simulink model "ofdm_txrx_mimo"  (Simulink 7.1)
settings: Solver=ode45  StartTime=0.0  StopTime=6e3  RelTol=1e-3
PreLoadFcn:
  | ofdm_rx_mimo_init
InitFcn:
  | ofdm_rx_mimo_init
StartFcn:
  | ofdm_rx_mimo_init
values from neighbouring files (symbols this model uses):
  BER_RAM_init_size = 2048    [ofdm_tx_mimo_init.m]
  CPLength = 16    [ofdm_tx_mimo_init.m]
  CRCPolynomial = hex2dec('04c11db7')    [ofdm_tx_mimo_init.m]
  freqOffset_track_filtCoef_i = hex2dec('2500')/2^32    [ofdm_rx_mimo_init.m]
  freqOffset_track_filtCoef_p = hex2dec('d0000')/2^32    [ofdm_rx_mimo_init.m]
  hi = zeros(1,length(cci))    [ofdm_rx_mimo_init.m]
  hr = zeros(1,length(ccr))    [ofdm_rx_mimo_init.m]
  max_CP_length = 16    [ofdm_tx_mimo_init.m]
  max_num_baseRateSymbols = 31    [ofdm_tx_mimo_init.m]
  max_num_subcarriers = 64    [ofdm_tx_mimo_init.m]
  max_num_trainingSymbols = 15    [ofdm_tx_mimo_init.m]
  max_numBytes = 16384    [ofdm_tx_mimo_init.m]
  max_OFDM_symbols = 2047    [ofdm_tx_mimo_init.m]
  modConstellation_qam16 = .75*[1 3 -1 -3]./3    [ofdm_tx_mimo_init.m]
  modConstellation_qam256 = 0.9375*[3 1 5 7 11 9 13 15 -3 -1 -5 -7 -11 -9 -13 -15]./15    [ofdm_tx_mimo_init.m]
  modConstellation_qam64 = 0.875*[3 1 5 7 -3 -1 -5 -7]./7    [ofdm_tx_mimo_init.m]
  modConstellation_qpsk = [1 -1]./sqrt(2)    [ofdm_tx_mimo_init.m]
  numBaseRateSymbols = 2    [ofdm_tx_mimo_init.m]
  numSubcarriers = 64    [ofdm_tx_mimo_init.m]
  numTrainingSymbols = 2    [ofdm_tx_mimo_init.m]
  pilot1_indicies = 7 + ( (64-7) * 2^16)    (= 3735559)    [ofdm_tx_mimo_init.m]
  pilot2_indicies = 21 + ( (64-21) * 2^16)    (= 2818069)    [ofdm_tx_mimo_init.m]
  pilotValue_neg = hex2dec('8000') + (2^16 * 0)    [ofdm_tx_mimo_init.m]
  pilotValue_pos = hex2dec('7FFF') + (2^16 * 0)    [ofdm_tx_mimo_init.m]
  pkt_crossCorr_thresh = 0.7    [ofdm_rx_mimo_init.m]
  pkt_energy_thresh = 0    [ofdm_rx_mimo_init.m]
  pkt_numPayloadBytes = 1400+42-6    (= 1436)    [ofdm_tx_mimo_init.m]
  pktByteNums = numHeaderBytes +  				byteIndex_numPayloadBytes(1)*2^8 +  				byteIndex_numPayloadBytes(2)*2^16 +  				byteIndex_simpleDynModMasks * 2^24    [ofdm_rx_mimo_init.m]
  PktDet_Delay = 26 + Symbol_Timing_Offset*2^7    (= 1050)    [ofdm_rx_mimo_init.m]
  preamble = 6*[0 shortsyms_10 longsyms_2]    [ofdm_tx_mimo_init.m]
  preamble_I = real(preamble)    [ofdm_tx_mimo_init.m]
  preamble_Q = imag(preamble)    [ofdm_tx_mimo_init.m]
  RAM_init_size = max_numBytes    (= 16384)    [ofdm_tx_mimo_init.m]
  reg_Interrupt_PktBuf_Control = reg_InterruptControl +  	1 * 2^16 +  	0 * 2^24    (= 65656)    [ofdm_rx_mimo_init.m]
  rx_controlBits = 1 * 2  		 + 1 * 4  		 + 0 * 8  		 + 1 * 16 * rx_SISO_Mode  		 + 1 * 32  		 + 1 * 64  		 + 0 * 128  		 + 1 * 256  		 + 0 * 512  		 + 1 * 1024  		 + 0 * 2048  		 + 1 * 4096  		 + 1 * 8192  		 + 0 * 16384  		 + 1 * 65536  		 + 1 * 131072  		 + 0 * 262144  		 + 1 * 524288    [ofdm_rx_mimo_init.m]
  Rx_PhaseNoiseTrack_K = 0.6094    [ofdm_rx_mimo_init.m]
  rx_postEq_scaling = round(rxScaling*2^11) * (1 + 2^16)    [ofdm_rx_mimo_init.m]
  RxDataScrambling_Seq = [40 198 78 63 82 173 102 245 48 111 172 115 147 230 216 93 72 65 62 2 205 242 122 90 128 83 105 97 73 10 5 252]    [ofdm_tx_mimo_init.m]
  simOnly_numSamples = length(preamble)+( (numSubcarriers+CPLength)*(numTrainingSymbols + numBaseRateSymbols + numFullRateSymbols/2) )    [ofdm_tx_mimo_init.m]
  train = [train train]    [ofdm_tx_mimo_init.m]
  tx_controlBits = (antB_preambleShift * 2^4) + (2^0 * tx_SISO_Mode) + 4*0 + 8*1    (= 73)    [ofdm_tx_mimo_init.m]
  TxDataScrambling_Seq = [40 198 78 63 82 173 102 245 48 111 172 115 147 230 216 93 72 65 62 2 205 242 122 90 128 83 105 97 73 10 5 252]    [ofdm_tx_mimo_init.m]
  txReg_symbolCounts = (2^16 * numFullRateSymbols) + (2^8 *numBaseRateSymbols ) + numTrainingSymbols    [ofdm_tx_mimo_init.m]
  TxRx_FFTScaling = bin2dec('010111') + (bin2dec('000101') * 2^6)    [ofdm_tx_mimo_init.m]
undefined symbols (not defined in the file or in its folder): AntA, AntB, BaseRate, CRC_table_gen, gcbh, intfilt, pktTiming_count_payloadStart, pktTiming_symbol_length, xlBlockLoadCallback, xlGetSimulinkPeriod
libraries: xbsIndex_r4 x4212, dspmlti4 x4, dspsigops x4, commchan3 x2, simulink x2, commrflib2 x2, dspsrcs4 x1
(part 3 of 43)

== system ofdm_txrx_mimo/Memory-mapped Registers ==
  Rx Registers : Subsystem
  Static Parameters (might be registers someday) : Subsystem
  Tx Registers : Subsystem
  TxRx Registers : Subsystem

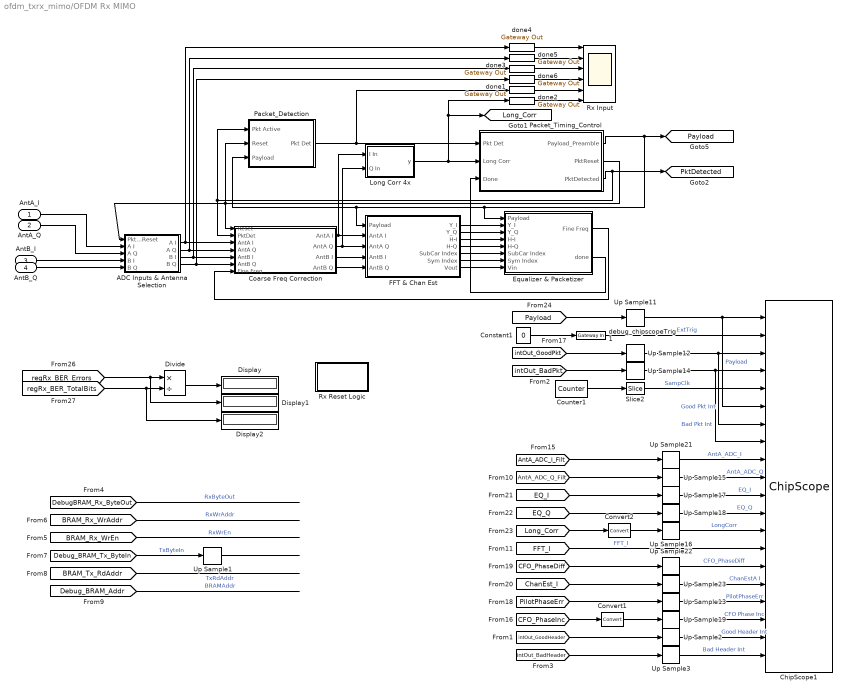
== system ofdm_txrx_mimo/OFDM Rx MIMO ==
  AntA_I : Inport  OutDataType=sfix(16)  OutScaling=2^0
  AntA_Q : Inport  Port=2  OutDataType=sfix(16)  OutScaling=2^0
  AntB_I : Inport  Port=3  OutDataType=sfix(16)  OutScaling=2^0
  AntB_Q : Inport  Port=4  OutDataType=sfix(16)  OutScaling=2^0
  ADC Inputs & Antenna Selection : Subsystem
  ChipScope1 : xbsIndex_r4/ChipScope  num_trig_ports=5  current_port=4  show_trig_port=0  match_units=1  match_type=Basic with edges  data_is_trigger=off  num_data_ports=15  data_depth=4096  SRL16s=on  match_type_t0=2  match_type_t1=2  match_type_t2=2  match_type_t3=2  match_type_t4=2  match_type_t5=1  match_type_t6=1  match_type_t7=1  match_type_t8=1  match_type_t9=1  match_type_t10=1  match_type_t11=1  match_type_t12=1  match_type_t13=1  match_type_t14=1  match_type_t15=1  match_units_t0=1  match_units_t1=1  match_units_t2=1  match_units_t3=1  match_units_t4=1  match_units_t5=1  match_units_t6=1  match_units_t7=1  match_units_t8=1  match_units_t9=1  match_units_t10=1  match_units_t11=1  match_units_t12=1  match_units_t13=1  match_units_t14=1  match_units_t15=1  dbl_ovrd=0  block_type=chipscope
      settings: infoedit="Enables run-time debugging and verification of signals by inserting ChipScope Pro ICON and ILA cores.<br><br>  Restrictions: <br>Only one ChipScope core can be instantiated in a System Generator design.This block cannot be used at the same time as JTAG hardware co-simulation.A design or subsystem containing a ChipScope block must have at least one output port."  block_version=VER_STRING_GOES_HERE
  Coarse Freq Correction : Subsystem
  Constant1 : Constant  Value=0  OutDataType=sfix(16)  OutScaling=2^0
  Convert1 : xbsIndex_r4/Convert  arith_type=Unsigned  n_bits=20  bin_pt=20  quantization=Truncate  overflow=Wrap  latency=0  dbl_ovrd=off  pipeline=off  block_type=convert
      settings: xl_area=[0 0 0 0 0 0 0]  block_version=VER_STRING_GOES_HERE
  Convert2 : xbsIndex_r4/Convert  arith_type=Unsigned  n_bits=14  bin_pt=0  quantization=Truncate  overflow=Wrap  latency=0  dbl_ovrd=off  pipeline=off  block_type=convert
      settings: xl_area=[0 0 0 0 0 0 0]  block_version=VER_STRING_GOES_HERE
  Counter1 : xbsIndex_r4/Counter  cnt_type=Free Running  cnt_to=Inf  operation=Up  start_count=0  cnt_by_val=1  arith_type=Unsigned  n_bits=2  bin_pt=0  load_pin=off  rst=off  en=off  explicit_period=on  period=1  dbl_ovrd=off  use_behavioral_HDL=off  use_rpm=on  implementation=Fabric  block_type=counter
      settings: xl_area=[5 10 0 10 0 0 0]  block_version=VER_STRING_GOES_HERE
  Display : Display
  Display1 : Display
  Display2 : Display
  Divide : Product  Inputs=*/  OutDataType=sfix(16)  OutScaling=2^-10
  Equalizer & Packetizer : Subsystem
  FFT & Chan Est : Subsystem
  Long Corr 4x : Subsystem
  Packet_Detection : Subsystem
  Packet_Timing_Control : Subsystem
  Rx Input : Scope  NumInputPorts=6
      settings: TimeRange=10000  YMin=-0.5~-0.4~-0.5~-0.4~0~0  YMax=0.5~0.4~0.5~0.4~1~7000  SaveName=ScopeData48
  Rx Reset Logic : Subsystem
  Slice2 : xbsIndex_r4/Slice  nbits=1  boolean_output=off  mode=Upper Bit Location + Width  bit1=0  base1=MSB of Input  bit0=0  base0=LSB of Input  dbl_ovrd=off  block_type=slice
      settings: infoedit="Extracts a given range of bits from each input sample and presents it at the output.  The output type is ordinarily unsigned with binary point at zero, but can be Boolean when the slice is one bit wide.<P><P>Hardware notes: In hardware this block costs nothing."  block_version=VER_STRING_GOES_HERE
  Up Sample{1..3,12..14,17..18,23} (9 alike) : xbsIndex_r4/Up Sample  sample_ratio=2  copy_samples=on  dbl_ovrd=off  block_type=usamp
      settings: infoedit="Up samples input data.  Inserted values  can be zeros or copies of the most recent input sample.<P><P>Hardware notes: No hardware is needed if inserted values are copies of the input sample; otherwise, a mux and single bit flip-flop are used."  block_version=VER_STRING_GOES_HERE
  Up Sample{11,15..16,19,21..22} (6 alike) : xbsIndex_r4/Up Sample  sample_ratio=4  copy_samples=on  dbl_ovrd=off  block_type=usamp
      settings: infoedit="Up samples input data.  Inserted values  can be zeros or copies of the most recent input sample.<P><P>Hardware notes: No hardware is needed if inserted values are copies of the input sample; otherwise, a mux and single bit flip-flop are used."  block_version=VER_STRING_GOES_HERE
  debug_chipscopeTrig1 : xbsIndex_r4/Gateway In  arith_type=Boolean  n_bits=14  bin_pt=13  quantization="Round  (unbiased: +/- Inf)"  overflow=Saturate  period=1  dbl_ovrd=off  timing_constraint=None  locs_specified=off  LOCs={}  block_type=gatewayin
      settings: infoedit="Gateway in block.  Converts inputs of type Simulink integer, double and fixed point to  Xilinx fixed point type.<P><P>Hardware notes:  In hardware these blocks become top level input ports."  block_version=VER_STRING_GOES_HERE
  done{1..6} (6 alike) : xbsIndex_r4/Gateway Out  hdl_port=off  timing_constraint=None  locs_specified=off  LOCs={}  block_type=gatewayout
      settings: infoedit="Gateway out block.  Converts Xilinx fixed point inputs into ouputs of type Simulink integer, double, or fixed point.<P><P>Hardware notes:  In hardware these blocks become top level output ports or are discarded, depending on how they are configured."  xl_area=[0 0 0 0 1 0 0]  block_version=VER_STRING_GOES_HERE
  tag scope: Long_Corr=global, Payload=global, PktDetected=global
  wires:
  Packet_Detection/1(Pkt Det) -> done1/1, Packet_Timing_Control/1(Pkt Det)
  Packet_Timing_Control/1(Payload_Preamble) -> Equalizer & Packetizer/1(Payload), FFT & Chan Est/1(Payload), Packet_Detection/3(Payload), [Payload]
  Long Corr 4x/1(y) -> Packet_Timing_Control/2(Long Corr), done2/1, [Long_Corr]
  done1/1 -> Rx Input/5
  done4/1 -> Rx Input/1
  done5/1 -> Rx Input/2
  done3/1 -> Rx Input/3
  done6/1 -> Rx Input/4
  Coarse Freq Correction/1("AntA I ") -> Long Corr 4x/1(I In), FFT & Chan Est/2(AntA I)
  Coarse Freq Correction/2("AntA Q ") -> Long Corr 4x/2(Q In), FFT & Chan Est/3(AntA Q)
  Coarse Freq Correction/3("AntB I ") -> FFT & Chan Est/4(AntB I)
  Coarse Freq Correction/4("AntB Q ") -> FFT & Chan Est/5(AntB Q)
  ADC Inputs & Antenna Selection/1(A I) -> Coarse Freq Correction/3(AntA I), done4/1
  ADC Inputs & Antenna Selection/2(A Q) -> Coarse Freq Correction/4(AntA Q), done5/1
  ADC Inputs & Antenna Selection/3(B I) -> Coarse Freq Correction/5(AntB I), done3/1
  ADC Inputs & Antenna Selection/4(B Q) -> Coarse Freq Correction/6(AntB Q), done6/1
  done2/1 -> Rx Input/6
  AntB_I/1 -> ADC Inputs & Antenna Selection/4("B I ")
  AntB_Q/1 -> ADC Inputs & Antenna Selection/5("B Q ")
  Equalizer & Packetizer/1(Fine Freq) -> Coarse Freq Correction/7(Fine Freq)
  Equalizer & Packetizer/2(done) -> Packet_Timing_Control/3(Done)
  FFT & Chan Est/1(Y_I) -> Equalizer & Packetizer/2(Y_I)
  FFT & Chan Est/2(Y_Q) -> Equalizer & Packetizer/3(Y_Q)
  FFT & Chan Est/3(H-I) -> Equalizer & Packetizer/4(H-I)
  FFT & Chan Est/4(H-Q) -> Equalizer & Packetizer/5(H-Q)
  FFT & Chan Est/5(SubCar Index) -> Equalizer & Packetizer/6(SubCar Index)
  FFT & Chan Est/6(Sym Index) -> Equalizer & Packetizer/7(Sym Index)
  FFT & Chan Est/7(Vout) -> Equalizer & Packetizer/8(Vin)
  Packet_Timing_Control/2(PktReset) -> Coarse Freq Correction/1(Reset), ADC Inputs & Antenna Selection/1(PktDetReset), Packet_Detection/2(Reset)
  Packet_Timing_Control/3(PktDetected) -> [PktDetected], Coarse Freq Correction/2(PktDet), Packet_Detection/1(Pkt Active)
  [Payload] -> Up Sample11/1
  [AntA_ADC_I_Filt] -> Up Sample21/1
  [EQ_I] -> Up Sample17/1
  [EQ_Q] -> Up Sample18/1
  [Long_Corr] -> Convert2/1
  [FFT_I] -> ChipScope1/14  "FFT_I"
  Constant1/1 -> debug_chipscopeTrig1/1
  Up Sample11/1 -> ChipScope1/6, ChipScope1/1  "Payload"
  Up Sample16/1 -> ChipScope1/13  "LongCorr"
  Up Sample17/1 -> ChipScope1/11  "EQ_I"
  Up Sample18/1 -> ChipScope1/12  "EQ_Q"
  Up Sample21/1 -> ChipScope1/9  "AntA_ADC_I"
  debug_chipscopeTrig1/1 -> ChipScope1/2  "ExtTrig"
  [intOut_GoodPkt] -> Up Sample12/1
  [intOut_BadPkt] -> Up Sample14/1
  Up Sample12/1 -> ChipScope1/7, ChipScope1/3  "Good Pkt Int"
  Up Sample14/1 -> ChipScope1/8, ChipScope1/4  "Bad Pkt Int"
  [AntA_ADC_Q_Filt] -> Up Sample15/1
  Up Sample15/1 -> ChipScope1/10  "AntA_ADC_Q"
  Counter1/1 -> Slice2/1
  Slice2/1 -> ChipScope1/5  "SampClk"
  Divide/1 -> Display/1
  [regRx_BER_Errors] -> Divide/1, Display1/1
  [regRx_BER_TotalBits] -> Divide/2, Display2/1
  [CFO_PhaseDiff] -> Up Sample22/1
  Up Sample22/1 -> ChipScope1/15  "CFO_PhaseDiff"
  [ChanEst_I] -> Up Sample23/1
  Up Sample23/1 -> ChipScope1/16  "ChanEstA I"
  Up Sample13/1 -> ChipScope1/17  "PilotPhaseErr"
  Up Sample19/1 -> ChipScope1/18  "CFO Phase Inc"
  [CFO_PhaseInc] -> Convert1/1
  [PilotPhaseErr] -> Up Sample13/1
  Convert1/1 -> Up Sample19/1
  [DebugBRAM_Rx_ByteOut] -> ?  "RxByteOut"
  [BRAM_Rx_WrAddr] -> ?  "RxWrAddr"
  [BRAM_Rx_WrEn] -> ?  "RxWrEn"
  [Debug_BRAM_Tx_ByteIn] -> Up Sample1/1  "TxByteIn"
  [BRAM_Tx_RdAddr] -> ?  "TxRdAddr"
  [Debug_BRAM_Addr] -> ?  "BRAMAddr"
  Up Sample1/1 -> ?
  Convert2/1 -> Up Sample16/1
  [intOut_GoodHeader] -> Up Sample2/1
  [intOut_BadHeader] -> Up Sample3/1
  Up Sample2/1 -> ChipScope1/19  "Good Header Int"
  Up Sample3/1 -> ChipScope1/20  "Bad Header Int"
  AntA_I/1 -> ADC Inputs & Antenna Selection/2("A I ")
  AntA_Q/1 -> ADC Inputs & Antenna Selection/3("A Q ")

== system ofdm_txrx_mimo/OFDM Tx MIMO ==
  Filter & DACs : Subsystem
  IFFT : Subsystem
  OutputBuffers : Subsystem
  OutputMuxes : Subsystem
  Training_Data : Subsystem  Orientation=left
  Tx Reset Logic : Subsystem
  Tx Scope : Scope  NumInputPorts=8
      settings: TimeRange=3000  YMin=-1~-1~-1~0.2375~0.2375~-1~-1~-1  YMax=1~1~1~0.2625~0.2625~1~1~1  SaveName=ScopeData30
  TxControl : Subsystem
  TxStart : Subsystem
  AntA I : Outport  OutDataType=sfix(16)  OutScaling=2^0
  AntA Q : Outport  Port=2  OutDataType=sfix(16)  OutScaling=2^0
  AntB I : Outport  Port=3  OutDataType=sfix(16)  OutScaling=2^0
  AntB Q : Outport  Port=4  OutDataType=sfix(16)  OutScaling=2^0
  wires:
  TxStart/1(Start) -> TxControl/1(StartTx), Tx Scope/1
  Training_Data/1(tx_Q) -> IFFT/2(xn_Q), Tx Scope/8
  TxControl/7(Start IFFT) -> IFFT/3(Start), Tx Scope/3
  IFFT/3(xk_I) -> OutputBuffers/2(I), Tx Scope/6
  IFFT/4(xk_Q) -> OutputBuffers/3(Q)
  IFFT/1(xn_Index) -> Training_Data/7(xn_index)
  IFFT/5(xk_Index) -> OutputBuffers/4(Xk_index)
  OutputBuffers/1(AntA_I) -> OutputMuxes/2(AntA I), Tx Scope/4
  OutputBuffers/2(AntA_Q) -> OutputMuxes/3(AntA Q), Tx Scope/5
  TxControl/5(Training_Data) -> Training_Data/2(train_pay)
  TxControl/6(CPn_Data) -> Training_Data/1(CPn_Data)
  TxControl/4(BaseRate_En) -> Training_Data/3(BaseRate_En)
  OutputBuffers/5(Vout) -> OutputMuxes/6(vin)
  Training_Data/2(tx_I) -> IFFT/1(xn_I), Tx Scope/7
  OutputBuffers/6(Pkt Done) -> OutputMuxes/7(Pkt Done), Training_Data/6(packet_done), TxControl/2(PktDone), Tx Scope/2
  OutputBuffers/3(AntB_I) -> OutputMuxes/4(AntB I)
  OutputBuffers/4(AntB_Q) -> OutputMuxes/5(AntB Q)
  TxControl/1(Preamble Index) -> OutputMuxes/1(Pream Index)
  IFFT/2(Vout) -> OutputBuffers/1(IFFT Vout)
  OutputMuxes/1(" AntA I") -> Filter & DACs/1(A_I)
  OutputMuxes/2(" AntA Q") -> Filter & DACs/2(A_Q)
  OutputMuxes/3(" AntB I") -> Filter & DACs/3(B_I)
  OutputMuxes/4(" AntB Q") -> Filter & DACs/4(B_Q)
  TxControl/3(FFT En) -> Training_Data/4(FFT En)
  Filter & DACs/1(" A_I") -> AntA I/1
  Filter & DACs/2(" A_Q") -> AntA Q/1
  Filter & DACs/3(" B_I") -> AntB I/1
  Filter & DACs/4(" B_Q") -> AntB Q/1
  TxControl/2(Start) -> Training_Data/5(Start)

== system ofdm_txrx_mimo/Pkt Buffer BRAM Interface ==
  BRAM_Addr : xbsIndex_r4/Gateway Out  hdl_port=on  timing_constraint=None  locs_specified=off  LOCs={}  block_type=gatewayout
      settings: infoedit="Gateway out block.  Converts Xilinx fixed point inputs into ouputs of type Simulink integer, double, or fixed point.<P><P>Hardware notes:  In hardware these blocks become top level output ports or are discarded, depending on how they are configured."  xl_area=[0, 0, 0, 0, 0, 0, 0]  block_version=VER_STRING_GOES_HERE
  BRAM_DataIn : xbsIndex_r4/Gateway In  arith_type=Unsigned  n_bits=64  bin_pt=0  quantization="Round  (unbiased: +/- Inf)"  overflow=Saturate  period=1  dbl_ovrd=off  timing_constraint=None  locs_specified=off  LOCs={}  block_type=gatewayin
      settings: infoedit="Gateway in block.  Converts inputs of type Simulink integer, double and fixed point to  Xilinx fixed point type.<P><P>Hardware notes:  In hardware these blocks become top level input ports."  block_version=VER_STRING_GOES_HERE
  BRAM_Dout : xbsIndex_r4/Gateway Out  hdl_port=on  timing_constraint=None  locs_specified=off  LOCs={}  block_type=gatewayout
      settings: infoedit="Gateway out block.  Converts Xilinx fixed point inputs into ouputs of type Simulink integer, double, or fixed point.<P><P>Hardware notes:  In hardware these blocks become top level output ports or are discarded, depending on how they are configured."  xl_area=[0, 0, 0, 0, 0, 0, 0]  block_version=VER_STRING_GOES_HERE
  BRAM_Reset : xbsIndex_r4/Gateway Out  hdl_port=on  timing_constraint=None  locs_specified=off  LOCs={}  block_type=gatewayout
      settings: infoedit="Gateway out block.  Converts Xilinx fixed point inputs into ouputs of type Simulink integer, double, or fixed point.<P><P>Hardware notes:  In hardware these blocks become top level output ports or are discarded, depending on how they are configured."  block_version=8.2.02
  BRAM_WEn : xbsIndex_r4/Gateway Out  hdl_port=on  timing_constraint=None  locs_specified=off  LOCs={}  block_type=gatewayout
      settings: infoedit="Gateway out block.  Converts Xilinx fixed point inputs into ouputs of type Simulink integer, double, or fixed point.<P><P>Hardware notes:  In hardware these blocks become top level output ports or are discarded, depending on how they are configured."  xl_area=[0, 0, 0, 0, 0, 0, 0]  block_version=VER_STRING_GOES_HERE
  Constant : xbsIndex_r4/Constant  arith_type=Unsigned  const=0  n_bits=1  bin_pt=0  explicit_period=on  period=1  equ=P=C  opselect=C  inp2=PCIN>>17  opr=+  inp1=P  carry=CIN  dbl_ovrd=off  block_type=constant
      settings: block_version=8.2.02
  Mux : xbsIndex_r4/Mux  inputs=2  en=off  latency=0  precision=Full  arith_type=Unsigned  n_bits=16  bin_pt=14  quantization=Truncate  overflow=Wrap  dbl_ovrd=off  block_type=mux
      settings: block_version=8.2.02
  PLB Pkt Buffer : Subsystem
  Pkt Buffer Addressing : Subsystem
  tag scope: BRAM_Tx_DIn=global, Debug_BRAM_Addr=global
  wires:
  PLB Pkt Buffer/1(Data) -> BRAM_DataIn/1
  [BRAM_Rx_DOut] -> BRAM_Dout/1
  [BRAM_Rx_WrEn] -> BRAM_WEn/1
  BRAM_DataIn/1 -> [BRAM_Tx_DIn]
  Constant/1 -> BRAM_Reset/1
  Mux/1 -> BRAM_Addr/1, [Debug_BRAM_Addr]
  [BRAM_Rx_AnyWrEn] -> Mux/1
  BRAM_Dout/1 -> PLB Pkt Buffer/1(BRAM_Dout)
  BRAM_WEn/1 -> PLB Pkt Buffer/2(WEn)
  BRAM_Addr/1 -> PLB Pkt Buffer/3(Addr)
  Pkt Buffer Addressing/1(Tx_RdAddr) -> Mux/2
  Pkt Buffer Addressing/2(Rx_WrAddr) -> Mux/3

== system ofdm_txrx_mimo/Workspace Transmitter ==
  From Workspace : FromWorkspace  VariableName=rxAntI  SampleTime=4  Interpolate=off  OutputAfterFinalValue=Cyclic repetition
  From Workspace1 : FromWorkspace  VariableName=rxAntQ  SampleTime=4  Interpolate=off  OutputAfterFinalValue=Cyclic repetition
  RXreset : Constant  Value=0  OutDataType=sfix(16)  OutScaling=2^0  SampleTime=4
  A_I : Outport  OutDataType=sfix(16)  OutScaling=2^0
  A_Q : Outport  Port=2  OutDataType=sfix(16)  OutScaling=2^0
  B_I : Outport  Port=3  OutDataType=sfix(16)  OutScaling=2^0
  B_Q : Outport  Port=4  OutDataType=sfix(16)  OutScaling=2^0
  wires:
  From Workspace/1 -> A_I/1
  From Workspace1/1 -> A_Q/1
  RXreset/1 -> B_I/1, B_Q/1

== system ofdm_txrx_mimo/"Channel"/Freq Offset ==
  note: Optional additive carrier
      | Models effects of DC-offsets at Tx/Rx
      | Set gain=0.15 for realistic offset
  In1 : Inport  OutDataType=sfix(16)  OutScaling=2^0
  In2 : Inport  Port=2  OutDataType=sfix(16)  OutScaling=2^0
  Gain : Gain  Orientation=up  Gain=0  ParameterDataType=sfix(16)  ParameterScaling=2^0  OutDataType=sfix(16)  OutScaling=2^0
  Product4 : Product  OutDataType=sfix(16)  OutScaling=2^0  RndMeth=Zero
  Product5 : Product  OutDataType=sfix(16)  OutScaling=2^0  RndMeth=Zero
  Sine Wave : dspsrcs4/Sine Wave  Amplitude=1  Frequency=freqOffset/4  Phase=0  SampleMode=Discrete  OutComplex=Complex  CompMethod=Trigonometric fcn  TableSize=Speed  SampleTime=1  SamplesPerFrame=1  dataType=double  wordLen=16  udDataType=sfix(16)  fracBitsMode=Best precision  numFracBits=15  ResetState=Restart at time zero
  Sum : Sum  Inputs=|++  OutDataType=sfix(16)  OutScaling=2^0
  Sum1 : Sum  Inputs=|++  OutDataType=sfix(16)  OutScaling=2^0
  Out1 : Outport  OutDataType=sfix(16)  OutScaling=2^0
  Out2 : Outport  Port=2  OutDataType=sfix(16)  OutScaling=2^0
  tag scope: freqOffset=global
  wires:
  Sine Wave/1 -> Product4/2, Product5/2, [freqOffset], Gain/1
  In1/1 -> Product4/1
  Product4/1 -> Sum/1
  In2/1 -> Product5/1
  Product5/1 -> Sum1/1
  Sum/1 -> Out1/1
  Sum1/1 -> Out2/1
  Gain/1 -> Sum1/2, Sum/2

== system ofdm_txrx_mimo/"Channel"/MIMO "Channel" ==
  In1 : Inport  OutDataType=sfix(16)  OutScaling=2^0
  In2 : Inport  Port=2  OutDataType=sfix(16)  OutScaling=2^0
  Add : Sum  OutDataType=sfix(16)  OutScaling=2^-10
  Add1 : Sum  OutDataType=sfix(16)  OutScaling=2^-10
  Constant : Constant  Value=h(1,1)  OutDataType=sfix(16)  OutScaling=2^0
  Constant1 : Constant  Value=h(2,1)  OutDataType=sfix(16)  OutScaling=2^0
  Constant2 : Constant  Value=h(1,2)  OutDataType=sfix(16)  OutScaling=2^0
  Constant3 : Constant  Value=h(2,2)  OutDataType=sfix(16)  OutScaling=2^0
  Display{,1..3} (4 alike) : Display
  Product{,1..3} (4 alike) : Product  OutDataType=sfix(16)  OutScaling=2^0  RndMeth=Zero
  Out1 : Outport  OutDataType=sfix(16)  OutScaling=2^0
  Out2 : Outport  Port=2  OutDataType=sfix(16)  OutScaling=2^0
  wires:
  Constant/1 -> Product/2, Display/1
  Product/1 -> Add/1
  In1/1 -> Product/1, Product2/1
  In2/1 -> Product1/2, Product3/2
  Constant1/1 -> Product1/1, Display1/1
  Product1/1 -> Add/2
  Constant2/1 -> Product2/2, Display2/1
  Product2/1 -> Add1/1
  Constant3/1 -> Product3/1, Display3/1
  Product3/1 -> Add1/2
  Add/1 -> Out1/1
  Add1/1 -> Out2/1

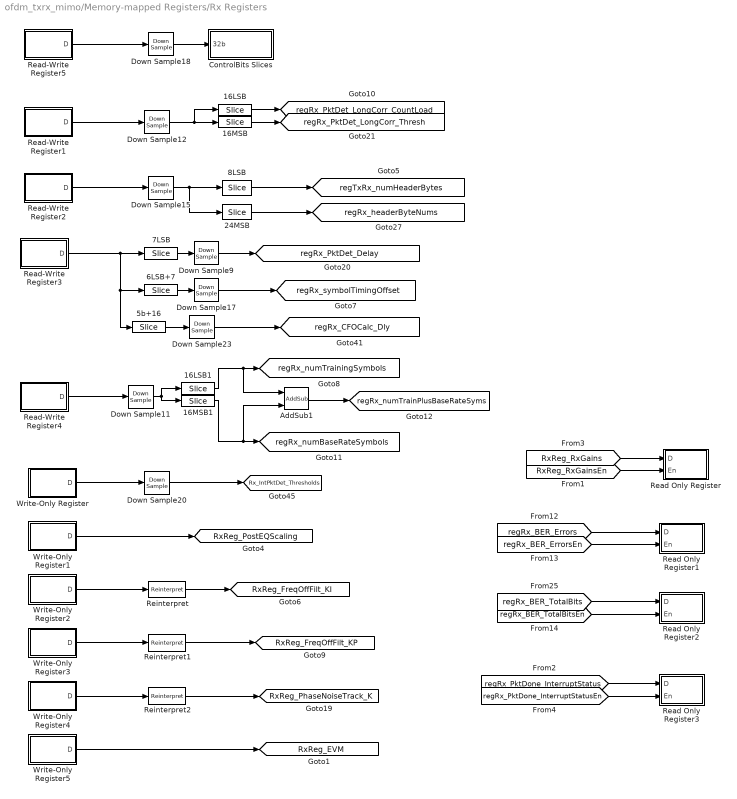
== system ofdm_txrx_mimo/Memory-mapped Registers/Rx Registers ==
  16LSB : xbsIndex_r4/Slice  nbits=16  boolean_output=off  mode=Lower Bit Location + Width  bit1=0  base1=MSB of Input  bit0=0  base0=LSB of Input  dbl_ovrd=off  block_type=slice
      settings: infoedit="Extracts a given range of bits from each input sample and presents it at the output.  The output type is ordinarily unsigned with binary point at zero, but can be Boolean when the slice is one bit wide.<P><P>Hardware notes: In hardware this block costs nothing."  block_version=VER_STRING_GOES_HERE
  16LSB1 : xbsIndex_r4/Slice  nbits=ceil(log2(max_num_trainingSymbols))  boolean_output=off  mode=Lower Bit Location + Width  bit1=0  base1=MSB of Input  bit0=0  base0=LSB of Input  dbl_ovrd=off  block_type=slice
      settings: infoedit="Extracts a given range of bits from each input sample and presents it at the output.  The output type is ordinarily unsigned with binary point at zero, but can be Boolean when the slice is one bit wide.<P><P>Hardware notes: In hardware this block costs nothing."  block_version=VER_STRING_GOES_HERE
  16MSB : xbsIndex_r4/Slice  nbits=16  boolean_output=off  mode=Lower Bit Location + Width  bit1=0  base1=MSB of Input  bit0=16  base0=LSB of Input  dbl_ovrd=off  block_type=slice
      settings: infoedit="Extracts a given range of bits from each input sample and presents it at the output.  The output type is ordinarily unsigned with binary point at zero, but can be Boolean when the slice is one bit wide.<P><P>Hardware notes: In hardware this block costs nothing."  block_version=VER_STRING_GOES_HERE
  16MSB1 : xbsIndex_r4/Slice  nbits=ceil(log2(max_num_baseRateSymbols))  boolean_output=off  mode=Lower Bit Location + Width  bit1=0  base1=MSB of Input  bit0=16  base0=LSB of Input  dbl_ovrd=off  block_type=slice
      settings: infoedit="Extracts a given range of bits from each input sample and presents it at the output.  The output type is ordinarily unsigned with binary point at zero, but can be Boolean when the slice is one bit wide.<P><P>Hardware notes: In hardware this block costs nothing."  block_version=VER_STRING_GOES_HERE
  24MSB : xbsIndex_r4/Slice  nbits=24  boolean_output=off  mode=Lower Bit Location + Width  bit1=0  base1=MSB of Input  bit0=8  base0=LSB of Input  dbl_ovrd=off  block_type=slice
      settings: infoedit="Extracts a given range of bits from each input sample and presents it at the output.  The output type is ordinarily unsigned with binary point at zero, but can be Boolean when the slice is one bit wide.<P><P>Hardware notes: In hardware this block costs nothing."  block_version=VER_STRING_GOES_HERE
  5b+16 : xbsIndex_r4/Slice  nbits=5  boolean_output=off  mode=Lower Bit Location + Width  bit1=0  base1=MSB of Input  bit0=16  base0=LSB of Input  dbl_ovrd=off  block_type=slice
      settings: infoedit="Extracts a given range of bits from each input sample and presents it at the output.  The output type is ordinarily unsigned with binary point at zero, but can be Boolean when the slice is one bit wide.<P><P>Hardware notes: In hardware this block costs nothing."  block_version=VER_STRING_GOES_HERE
  6LSB+7 : xbsIndex_r4/Slice  nbits=6  boolean_output=off  mode=Lower Bit Location + Width  bit1=0  base1=MSB of Input  bit0=7  base0=LSB of Input  dbl_ovrd=off  block_type=slice
      settings: infoedit="Extracts a given range of bits from each input sample and presents it at the output.  The output type is ordinarily unsigned with binary point at zero, but can be Boolean when the slice is one bit wide.<P><P>Hardware notes: In hardware this block costs nothing."  block_version=VER_STRING_GOES_HERE
  7LSB : xbsIndex_r4/Slice  nbits=7  boolean_output=off  mode=Lower Bit Location + Width  bit1=0  base1=MSB of Input  bit0=0  base0=LSB of Input  dbl_ovrd=off  block_type=slice
      settings: infoedit="Extracts a given range of bits from each input sample and presents it at the output.  The output type is ordinarily unsigned with binary point at zero, but can be Boolean when the slice is one bit wide.<P><P>Hardware notes: In hardware this block costs nothing."  block_version=VER_STRING_GOES_HERE
  "8LSB " : xbsIndex_r4/Slice  nbits=8  boolean_output=off  mode=Lower Bit Location + Width  bit1=0  base1=MSB of Input  bit0=0  base0=LSB of Input  dbl_ovrd=off  block_type=slice
      settings: infoedit="Extracts a given range of bits from each input sample and presents it at the output.  The output type is ordinarily unsigned with binary point at zero, but can be Boolean when the slice is one bit wide.<P><P>Hardware notes: In hardware this block costs nothing."  block_version=VER_STRING_GOES_HERE
  AddSub1 : xbsIndex_r4/AddSub  mode=Addition  use_carryin=off  use_carryout=off  en=off  latency=0  precision=User Defined  arith_type=Unsigned  n_bits=1 + ceil(log2(max_num_trainingSymbols + max_num_baseRateSymbols))  bin_pt=0  quantization=Truncate  overflow=Wrap  dbl_ovrd=off  use_behavioral_HDL=on  pipelined=off  use_rpm=on  hw_selection=Fabric  block_type=addsub
      settings: xl_area=[0, 0, 0, 0, 0, 0, 0]  block_version=VER_STRING_GOES_HERE
  ControlBits Slices : Subsystem
  Down Sample{11,15,17..18} (4 alike) : xbsIndex_r4/Down Sample  sample_ratio=2  sample_phase="Last Value of Frame  (most efficient)"  en=off  latency=1  dbl_ovrd=off  block_type=dsamp
      settings: block_version=VER_STRING_GOES_HERE
  Down Sample{9,12,20,23} (4 alike) : xbsIndex_r4/Down Sample  sample_ratio=4  sample_phase="Last Value of Frame  (most efficient)"  en=off  latency=1  dbl_ovrd=off  block_type=dsamp
      settings: block_version=VER_STRING_GOES_HERE
  Read Only Register : Subsystem  AncestorBlock=|  mask{regName='Rx_Gains'}
      | WARP_Blockset/Register Wrappers
      | (Sysgen 10.1+)/Read Only Register
      mask prompts: regName "Register Name"
      mask help:
      | This block is a wrapper for System Generator's To Register shared memory block. This register is designed for use with WARP's sysgen2opb tool.
      |
      | Note: The Regisger Name paramter below must be unique for every instance of Read-Write and Read-Only registers in your system.
      mask init:
      | regBlock = find_system(gcb, 'LookUnderMasks', 'all', 'FollowLinks', 'on',   'block_type', 'toreg');
      | 
      | if(length(regBlock) > 0)
      | 
      |     %Set the shared memory name
      |     set_param(regBlock{1}, 'shared_memory_name', regName);
      | 
      | 	set_param(regBlock{1}, 'explicit_data_type', 'on')
      |     set_param(regBlock{1}, 'arith_type', 'unsigned');
      | 	set_param(regBlock{1}, 'ownership', 'Locally owned and initialized')
      | 
      | 	set_param(regBlock{1}, 'n_bits', '32');
      | 	set_param(regBlock{1}, 'bin_pt', '0');
      | 	set_param(regBlock{1}, 'init', '0');
      | end
      settings: AttributesFormatString=RO Reg: %<regName>
  Read Only Register1 : Subsystem  AncestorBlock=|  mask{regName='Rx_BER_Errors'}
      | WARP_Blockset/Register Wrappers
      | (Sysgen 10.1+)/Read Only Register
      mask prompts: regName "Register Name"
      mask help:
      | This block is a wrapper for System Generator's To Register shared memory block. This register is designed for use with WARP's sysgen2opb tool.
      |
      | Note: The Regisger Name paramter below must be unique for every instance of Read-Write and Read-Only registers in your system.
      mask init: same as ofdm_txrx_mimo/Memory-mapped Registers/Rx Registers/Read Only Register
      settings: AttributesFormatString=RO Reg: %<regName>
  Read Only Register2 : Subsystem  AncestorBlock=|  mask{regName='Rx_BER_TotalBits'}
      | WARP_Blockset/Register Wrappers
      | (Sysgen 10.1+)/Read Only Register
      mask prompts: regName "Register Name"
      mask help:
      | This block is a wrapper for System Generator's To Register shared memory block. This register is designed for use with WARP's sysgen2opb tool.
      |
      | Note: The Regisger Name paramter below must be unique for every instance of Read-Write and Read-Only registers in your system.
      mask init: same as ofdm_txrx_mimo/Memory-mapped Registers/Rx Registers/Read Only Register
      settings: AttributesFormatString=RO Reg: %<regName>
  Read Only Register3 : Subsystem  AncestorBlock=|  mask{regName='Rx_pktDone_interruptStatus'}
      | WARP_Blockset/Register Wrappers
      | (Sysgen 10.1+)/Read Only Register
      mask prompts: regName "Register Name"
      mask help:
      | This block is a wrapper for System Generator's To Register shared memory block. This register is designed for use with WARP's sysgen2opb tool.
      |
      | Note: The Regisger Name paramter below must be unique for every instance of Read-Write and Read-Only registers in your system.
      mask init: same as ofdm_txrx_mimo/Memory-mapped Registers/Rx Registers/Read Only Register
      settings: AttributesFormatString=RO Reg: %<regName>
  Read-Write Register1 : Subsystem  AncestorBlock=|  mask{regName='Rx_PktDet_LongCorr_Params', out_dataType=Unsigned, out_numBits=32, out_binaryPt=0, initValue=251-16 + (2^16 * 3000), sampPeriod=1}
      | WARP_Blockset/Register Wrappers
      | (Sysgen 10.1+)/Read-Write Register
      mask prompts: regName "Register Name"; out_dataType "Output Type"; out_numBits "Output Num Bits"; out_binaryPt "Output Binary Point"; initValue "Initial Value"; sampPeriod "Sample Period"
      mask help:
      | This block is a wrapper for two registers from the System Generator Shared Memory blockset:
      | a) A "From Register" block, which provides a write-only register for the host processor
      | b) A "To Register" block, which provides a read-only register for the host processor
      |
      | Note: The Regisger Name paramter below must be unique for every instance of shared-memory registers in your system. System Generator will throw an error if duplicate names are found.
      mask init:
      | wo_regBlock = find_system(gcb, 'LookUnderMasks', 'all', 'FollowLinks', 'on', 'block_type', 'fromreg');
      | ro_regBlock = find_system(gcb, 'LookUnderMasks', 'all', 'FollowLinks', 'on', 'block_type', 'toreg');
      | 
      | if(length(wo_regBlock)>0)
      | 
      | 	%Set the shared memory name
      | 	set_param(wo_regBlock{1}, 'shared_memory_name', ['''' strcat(regName(2:end-1), '_w') '''']);
      | 
      | 	set_param(wo_regBlock{1}, 'arith_type', sprintf('%s', out_dataType));
      | 	set_param(wo_regBlock{1}, 'ownership', 'Locally owned and initialized')
      | 
      | 	set_param(wo_regBlock{1}, 'n_bits', num2str(out_numBits));
      | 	set_param(wo_regBlock{1}, 'bin_pt', num2str(out_binaryPt));
      | 	set_param(wo_regBlock{1}, 'init', num2str(initValue));
      | end
      | 
      | if(length(ro_regBlock)>0)
      | 
      | 	%Set the shared memory name
      | 	set_param(ro_regBlock{1}, 'shared_memory_name', ['''' strcat(regName(2:end-1), '_r') '''']);
      | 
      | 	set_param(ro_regBlock{1}, 'explicit_data_type', 'on')
      |     set_param(ro_regBlock{1}, 'arith_type', sprintf('%s', out_dataType));
      | 	set_param(ro_regBlock{1}, 'ownership', 'Locally owned and initialized')
      | 
      | 	set_param(ro_regBlock{1}, 'n_bits', num2str(out_numBits));
      | 	set_param(ro_regBlock{1}, 'bin_pt', num2str(out_binaryPt));
      | 	set_param(ro_regBlock{1}, 'init', num2str(initValue));
      | end
      settings: AttributesFormatString=RW Reg: %<regName>
      mask StyleString: edit,popup(Unsigned|Signed  (2's comp)),edit,edit,edit,edit
  Read-Write Register2 : Subsystem  AncestorBlock=|  mask{regName='Rx_pktByteNums', out_dataType=Unsigned, out_numBits=32, out_binaryPt=0, initValue=pktByteNums, sampPeriod=1}
      | WARP_Blockset/Register Wrappers
      | (Sysgen 10.1+)/Read-Write Register
      mask prompts: regName "Register Name"; out_dataType "Output Type"; out_numBits "Output Num Bits"; out_binaryPt "Output Binary Point"; initValue "Initial Value"; sampPeriod "Sample Period"
      mask help:
      | This block is a wrapper for two registers from the System Generator Shared Memory blockset:
      | a) A "From Register" block, which provides a write-only register for the host processor
      | b) A "To Register" block, which provides a read-only register for the host processor
      |
      | Note: The Regisger Name paramter below must be unique for every instance of shared-memory registers in your system. System Generator will throw an error if duplicate names are found.
      mask init: same as ofdm_txrx_mimo/Memory-mapped Registers/Rx Registers/Read-Write Register1
      settings: AttributesFormatString=RW Reg: %<regName>
      mask StyleString: edit,popup(Unsigned|Signed  (2's comp)),edit,edit,edit,edit
  Read-Write Register3 : Subsystem  AncestorBlock=|  mask{regName='Rx_PktDet_Delay', out_dataType=Unsigned, out_numBits=32, out_binaryPt=0, initValue=PktDet_Delay, sampPeriod=1}
      | WARP_Blockset/Register Wrappers
      | (Sysgen 10.1+)/Read-Write Register
      mask prompts: regName "Register Name"; out_dataType "Output Type"; out_numBits "Output Num Bits"; out_binaryPt "Output Binary Point"; initValue "Initial Value"; sampPeriod "Sample Period"
      mask help:
      | This block is a wrapper for two registers from the System Generator Shared Memory blockset:
      | a) A "From Register" block, which provides a write-only register for the host processor
      | b) A "To Register" block, which provides a read-only register for the host processor
      |
      | Note: The Regisger Name paramter below must be unique for every instance of shared-memory registers in your system. System Generator will throw an error if duplicate names are found.
      mask init: same as ofdm_txrx_mimo/Memory-mapped Registers/Rx Registers/Read-Write Register1
      settings: AttributesFormatString=RW Reg: %<regName>
      mask StyleString: edit,popup(Unsigned|Signed  (2's comp)),edit,edit,edit,edit
  Read-Write Register4 : Subsystem  AncestorBlock=|  mask{regName='Rx_OFDM_SymbolCounts', out_dataType=Unsigned, out_numBits=32, out_binaryPt=0, initValue=(2^16 * numBaseRateSymbols) + numTrainingSymbols, sampPeriod=1}
      | WARP_Blockset/Register Wrappers
      | (Sysgen 10.1+)/Read-Write Register
      mask prompts: regName "Register Name"; out_dataType "Output Type"; out_numBits "Output Num Bits"; out_binaryPt "Output Binary Point"; initValue "Initial Value"; sampPeriod "Sample Period"
      mask help:
      | This block is a wrapper for two registers from the System Generator Shared Memory blockset:
      | a) A "From Register" block, which provides a write-only register for the host processor
      | b) A "To Register" block, which provides a read-only register for the host processor
      |
      | Note: The Regisger Name paramter below must be unique for every instance of shared-memory registers in your system. System Generator will throw an error if duplicate names are found.
      mask init: same as ofdm_txrx_mimo/Memory-mapped Registers/Rx Registers/Read-Write Register1
      settings: AttributesFormatString=RW Reg: %<regName>
      mask StyleString: edit,popup(Unsigned|Signed  (2's comp)),edit,edit,edit,edit
  Read-Write Register5 : Subsystem  AncestorBlock=|  mask{regName='Rx_ControlBits', out_dataType=Unsigned, out_numBits=32, out_binaryPt=0, initValue=rx_controlBits, sampPeriod=1}
      | WARP_Blockset/Register Wrappers
      | (Sysgen 10.1+)/Read-Write Register
      mask prompts: regName "Register Name"; out_dataType "Output Type"; out_numBits "Output Num Bits"; out_binaryPt "Output Binary Point"; initValue "Initial Value"; sampPeriod "Sample Period"
      mask help:
      | This block is a wrapper for two registers from the System Generator Shared Memory blockset:
      | a) A "From Register" block, which provides a write-only register for the host processor
      | b) A "To Register" block, which provides a read-only register for the host processor
      |
      | Note: The Regisger Name paramter below must be unique for every instance of shared-memory registers in your system. System Generator will throw an error if duplicate names are found.
      mask init: same as ofdm_txrx_mimo/Memory-mapped Registers/Rx Registers/Read-Write Register1
      settings: AttributesFormatString=RW Reg: %<regName>
      mask StyleString: edit,popup(Unsigned|Signed  (2's comp)),edit,edit,edit,edit
  Reinterpret : xbsIndex_r4/Reinterpret  force_arith_type=on  arith_type=Unsigned  force_bin_pt=on  bin_pt=32  block_type=reinterpret
      settings: infoedit="Changes signal type without altering the binary representation.   You can changed the signal between signed and unsigned, and relocate the binary point.<P><P>Hardware notes: In hardware this block costs nothing.<P><P>Example:  Suppose the input is 6 bits wide, signed, with 2 fractional bits, and the output is forced to unsigned with 0 fractional bits.  Then an input of -2.0 (1110.00 in binary 2's complement) becomes an output of 56 (111000 in binary)."  block_version=10.1
  Reinterpret1 : xbsIndex_r4/Reinterpret  force_arith_type=on  arith_type=Unsigned  force_bin_pt=on  bin_pt=32  block_type=reinterpret
      settings: infoedit="Changes signal type without altering the binary representation.   You can changed the signal between signed and unsigned, and relocate the binary point.<P><P>Hardware notes: In hardware this block costs nothing.<P><P>Example:  Suppose the input is 6 bits wide, signed, with 2 fractional bits, and the output is forced to unsigned with 0 fractional bits.  Then an input of -2.0 (1110.00 in binary 2's complement) becomes an output of 56 (111000 in binary)."  block_version=10.1
  Reinterpret2 : xbsIndex_r4/Reinterpret  force_arith_type=on  arith_type=Unsigned  force_bin_pt=on  bin_pt=26  block_type=reinterpret
      settings: infoedit="Changes signal type without altering the binary representation.   You can changed the signal between signed and unsigned, and relocate the binary point.<P><P>Hardware notes: In hardware this block costs nothing.<P><P>Example:  Suppose the input is 6 bits wide, signed, with 2 fractional bits, and the output is forced to unsigned with 0 fractional bits.  Then an input of -2.0 (1110.00 in binary 2's complement) becomes an output of 56 (111000 in binary)."  block_version=10.1
  Write-Only Register : Subsystem  AncestorBlock=|  mask{regName='Rx_pktDet_Tresholds', out_dataType=Unsigned, out_numBits=32, out_binaryPt=0, initValue=(2^8 * pkt_energy_thresh) + pkt_crossCorr_thresh, sampPeriod=1}
      | WARP_Blockset/Register Wrappers
      | (Sysgen 10.1+)/Write-Only Register
      mask prompts: regName "Register Name"; out_dataType "Output Type"; out_numBits "Output Num Bits"; out_binaryPt "Output Binary Point"; initValue "Initial Value"; sampPeriod "Sample Period"
      mask help:
      | This block is a wrapper for System Generator's "From Register" shared memory block.
      |
      | Note: The Regisger Name paramter below must be unique for every instance of shared-memory registers in your system. System Generator will throw an error if duplicate names are found.
      mask init:
      | regBlock = find_system(gcb, 'LookUnderMasks', 'all', 'FollowLinks', 'on', 'block_type', 'fromreg');
      | 
      | if(length(regBlock)>0)
      | 
      | 	%Set the shared memory name
      | 
      |     set_param(regBlock{1}, 'shared_memory_name', ['''' strcat(regName(2:end-1), '_w') '''']);
      | 
      | 	set_param(regBlock{1}, 'arith_type', sprintf('%s', out_dataType));
      | 	set_param(regBlock{1}, 'ownership', 'Locally owned and initialized')
      | 
      | 	set_param(regBlock{1}, 'n_bits', num2str(out_numBits));
      | 	set_param(regBlock{1}, 'bin_pt', num2str(out_binaryPt));
      | 	set_param(regBlock{1}, 'init', num2str(initValue));
      | end
      settings: AttributesFormatString=RW Reg: %<regName>
      mask StyleString: edit,popup(Unsigned|Signed  (2's comp)),edit,edit,edit,edit
  Write-Only Register1 : Subsystem  AncestorBlock=|  mask{regName='Rx_Constellation_Scaling', out_dataType=Unsigned, out_numBits=32, out_binaryPt=0, initValue=rx_postEq_scaling, sampPeriod=1}
      | WARP_Blockset/Register Wrappers
      | (Sysgen 10.1+)/Write-Only Register
      mask prompts: regName "Register Name"; out_dataType "Output Type"; out_numBits "Output Num Bits"; out_binaryPt "Output Binary Point"; initValue "Initial Value"; sampPeriod "Sample Period"
      mask help:
      | This block is a wrapper for System Generator's "From Register" shared memory block.
      |
      | Note: The Regisger Name paramter below must be unique for every instance of shared-memory registers in your system. System Generator will throw an error if duplicate names are found.
      mask init: same as ofdm_txrx_mimo/Memory-mapped Registers/Rx Registers/Write-Only Register
      settings: AttributesFormatString=RW Reg: %<regName>
      mask StyleString: edit,popup(Unsigned|Signed  (2's comp)),edit,edit,edit,edit
  Write-Only Register2 : Subsystem  AncestorBlock=|  mask{regName='Rx_FreqOffFilt_B_KI', out_dataType=Unsigned, out_numBits=32, out_binaryPt=0, initValue=freqOffset_track_filtCoef_i * 2^32, sampPeriod=1}
      | WARP_Blockset/Register Wrappers
      | (Sysgen 10.1+)/Write-Only Register
      mask prompts: regName "Register Name"; out_dataType "Output Type"; out_numBits "Output Num Bits"; out_binaryPt "Output Binary Point"; initValue "Initial Value"; sampPeriod "Sample Period"
      mask help:
      | This block is a wrapper for System Generator's "From Register" shared memory block.
      |
      | Note: The Regisger Name paramter below must be unique for every instance of shared-memory registers in your system. System Generator will throw an error if duplicate names are found.
      mask init: same as ofdm_txrx_mimo/Memory-mapped Registers/Rx Registers/Write-Only Register
      settings: AttributesFormatString=RW Reg: %<regName>
      mask StyleString: edit,popup(Unsigned|Signed  (2's comp)),edit,edit,edit,edit
  Write-Only Register3 : Subsystem  AncestorBlock=|  mask{regName='Rx_FreqOffFilt_B_KP', out_dataType=Unsigned, out_numBits=32, out_binaryPt=0, initValue=freqOffset_track_filtCoef_p * 2^32, sampPeriod=1}
      | WARP_Blockset/Register Wrappers
      | (Sysgen 10.1+)/Write-Only Register
      mask prompts: regName "Register Name"; out_dataType "Output Type"; out_numBits "Output Num Bits"; out_binaryPt "Output Binary Point"; initValue "Initial Value"; sampPeriod "Sample Period"
      mask help:
      | This block is a wrapper for System Generator's "From Register" shared memory block.
      |
      | Note: The Regisger Name paramter below must be unique for every instance of shared-memory registers in your system. System Generator will throw an error if duplicate names are found.
      mask init: same as ofdm_txrx_mimo/Memory-mapped Registers/Rx Registers/Write-Only Register
      settings: AttributesFormatString=RW Reg: %<regName>
      mask StyleString: edit,popup(Unsigned|Signed  (2's comp)),edit,edit,edit,edit
  Write-Only Register4 : Subsystem  AncestorBlock=|  mask{regName='Rx_PhaseNoiseTrack_K', out_dataType=Unsigned, out_numBits=32, out_binaryPt=0, initValue=Rx_PhaseNoiseTrack_K * 2^26, sampPeriod=1}
      | WARP_Blockset/Register Wrappers
      | (Sysgen 10.1+)/Write-Only Register
      mask prompts: regName "Register Name"; out_dataType "Output Type"; out_numBits "Output Num Bits"; out_binaryPt "Output Binary Point"; initValue "Initial Value"; sampPeriod "Sample Period"
      mask help:
      | This block is a wrapper for System Generator's "From Register" shared memory block.
      |
      | Note: The Regisger Name paramter below must be unique for every instance of shared-memory registers in your system. System Generator will throw an error if duplicate names are found.
      mask init: same as ofdm_txrx_mimo/Memory-mapped Registers/Rx Registers/Write-Only Register
      settings: AttributesFormatString=RW Reg: %<regName>
      mask StyleString: edit,popup(Unsigned|Signed  (2's comp)),edit,edit,edit,edit
  Write-Only Register5 : Subsystem  AncestorBlock=|  mask{regName='Rx_EVM', out_dataType=Unsigned, out_numBits=7, out_binaryPt=0, initValue=20, sampPeriod=1}
      | WARP_Blockset/Register Wrappers
      | (Sysgen 10.1+)/Write-Only Register
      mask prompts: regName "Register Name"; out_dataType "Output Type"; out_numBits "Output Num Bits"; out_binaryPt "Output Binary Point"; initValue "Initial Value"; sampPeriod "Sample Period"
      mask help:
      | This block is a wrapper for System Generator's "From Register" shared memory block.
      |
      | Note: The Regisger Name paramter below must be unique for every instance of shared-memory registers in your system. System Generator will throw an error if duplicate names are found.
      mask init: same as ofdm_txrx_mimo/Memory-mapped Registers/Rx Registers/Write-Only Register
      settings: AttributesFormatString=RW Reg: %<regName>
      mask StyleString: edit,popup(Unsigned|Signed  (2's comp)),edit,edit,edit,edit
  tag scope: RxReg_EVM=global, RxReg_FreqOffFilt_KI=global, RxReg_FreqOffFilt_KP=global, RxReg_PhaseNoiseTrack_K=global, RxReg_PostEQScaling=global, Rx_IntPktDet_Thresholds=global, regRx_CFOCalc_Dly=global, regRx_PktDet_Delay=global, regRx_PktDet_LongCorr_CountLoad=global, regRx_PktDet_LongCorr_Thresh=global, regRx_headerByteNums=global, regRx_numBaseRateSymbols=global, regRx_numTrainPlusBaseRateSyms=global, regRx_numTrainingSymbols=global, regRx_symbolTimingOffset=global, regTxRx_numHeaderBytes=global
  wires:
  Read-Write Register5/1(D) -> Down Sample18/1
  Read-Write Register2/1(D) -> Down Sample15/1
  AddSub1/1 -> [regRx_numTrainPlusBaseRateSyms]
  Read-Write Register3/1(D) -> 7LSB/1, 6LSB+7/1, 5b+16/1
  Down Sample9/1 -> [regRx_PktDet_Delay]
  Down Sample15/1 -> 24MSB/1, "8LSB "/1
  16LSB1/1 -> [regRx_numTrainingSymbols], AddSub1/1
  16MSB1/1 -> [regRx_numBaseRateSymbols], AddSub1/2
  16MSB/1 -> [regRx_PktDet_LongCorr_Thresh]
  16LSB/1 -> [regRx_PktDet_LongCorr_CountLoad]
  Read-Write Register1/1(D) -> Down Sample12/1
  Down Sample12/1 -> 16MSB/1, 16LSB/1
  Read-Write Register4/1(D) -> Down Sample11/1
  Down Sample11/1 -> 16MSB1/1, 16LSB1/1
  24MSB/1 -> [regRx_headerByteNums]
  7LSB/1 -> Down Sample9/1
  6LSB+7/1 -> Down Sample17/1
  Down Sample17/1 -> [regRx_symbolTimingOffset]
  Down Sample23/1 -> [regRx_CFOCalc_Dly]
  5b+16/1 -> Down Sample23/1
  Write-Only Register/1(D) -> Down Sample20/1
  Down Sample20/1 -> [Rx_IntPktDet_Thresholds]
  Down Sample18/1 -> ControlBits Slices/1(32b)
  "8LSB "/1 -> [regTxRx_numHeaderBytes]
  [RxReg_RxGains] -> Read Only Register/1(D)
  [RxReg_RxGainsEn] -> Read Only Register/2(En)
  Write-Only Register1/1(D) -> [RxReg_PostEQScaling]
  Write-Only Register3/1(D) -> Reinterpret1/1
  Write-Only Register2/1(D) -> Reinterpret/1
  Write-Only Register4/1(D) -> Reinterpret2/1
  [regRx_BER_TotalBitsEn] -> Read Only Register2/2(En)
  [regRx_BER_Errors] -> Read Only Register1/1(D)
  [regRx_BER_TotalBits] -> Read Only Register2/1(D)
  [regRx_BER_ErrorsEn] -> Read Only Register1/2(En)
  [regRx_PktDone_InterruptStatus] -> Read Only Register3/1(D)
  [regRx_PktDone_InterruptStatusEn] -> Read Only Register3/2(En)
  Reinterpret/1 -> [RxReg_FreqOffFilt_KI]
  Reinterpret1/1 -> [RxReg_FreqOffFilt_KP]
  Reinterpret2/1 -> [RxReg_PhaseNoiseTrack_K]
  Write-Only Register5/1(D) -> [RxReg_EVM]
